simulink model "AirDirecCtrlMgr"  (R2022b) (continued)
== system AirDirecCtrlMgr == (continued)
  ClimaCfgForVgaMotorPrsntAtRowFirstSideLe_ClimaCfgForVgaMotorPrsntAtRowFirstSideLe/1 -> AirDirecCtrlMgr_Runnable_sys/4(ClimaCfgForVgaMotorPrsntAtRowFirstSideLe_ClimaCfgForVgaMotorPrsntAtRowFirstSideLe_read)
  ClimaCfgForVgaMotorPrsntAtRowFirstSideRi_ClimaCfgForVgaMotorPrsntAtRowFirstSideRi/1 -> AirDirecCtrlMgr_Runnable_sys/5(ClimaCfgForVgaMotorPrsntAtRowFirstSideRi_ClimaCfgForVgaMotorPrsntAtRowFirstSideRi_read)
  ClimaCfgForVgaMotorPrsntAtRowSecSideLe_ClimaCfgForVgaMotorPrsntAtRowSecSideLe/1 -> AirDirecCtrlMgr_Runnable_sys/6(ClimaCfgForVgaMotorPrsntAtRowSecSideLe_ClimaCfgForVgaMotorPrsntAtRowSecSideLe_read)
  ClimaCfgForVgaMotorPrsntAtRowSecSideRi_ClimaCfgForVgaMotorPrsntAtRowSecSideRi/1 -> AirDirecCtrlMgr_Runnable_sys/7(ClimaCfgForVgaMotorPrsntAtRowSecSideRi_ClimaCfgForVgaMotorPrsntAtRowSecSideRi_read)
  ClimaCtrlActvNgcc_ClimaCtrlActv/1 -> AirDirecCtrlMgr_Runnable_sys/8(ClimaCtrlActvNgcc_ClimaCtrlActv_read)
  DrvrHdPosnDetn_DrvrHdPosnDetn/1 -> AirDirecCtrlMgr_Runnable_sys/9(DrvrHdPosnDetn_DrvrHdPosnDetn_read)
  FrntHvacBlowerSts_FrntHvacBlowerSts/1 -> AirDirecCtrlMgr_Runnable_sys/10(FrntHvacBlowerSts_FrntHvacBlowerSts_read)
  FrntVGAerror_FrntVGAerror/1 -> AirDirecCtrlMgr_Runnable_sys/11(FrntVGAerror_FrntVGAerror_read)
  HmiElecAirAdpvReq_HmiElecAirAdpvReq/1 -> AirDirecCtrlMgr_Runnable_sys/12(HmiElecAirAdpvReq_HmiElecAirAdpvReq_read)
  HmiElecAirDirCrtlReq_HmiElecAirDirCrtlReq/1 -> AirDirecCtrlMgr_Runnable_sys/13(HmiElecAirDirCrtlReq_HmiElecAirDirCrtlReq_read)
  HmiElecAirDirModReq_HmiElecAirDirModReq/1 -> AirDirecCtrlMgr_Runnable_sys/14(HmiElecAirDirModReq_HmiElecAirDirModReq_read)
  HmiElecAirDrvrSwtReq_HmiElecAirDrvrSwtReq/1 -> AirDirecCtrlMgr_Runnable_sys/15(HmiElecAirDrvrSwtReq_HmiElecAirDrvrSwtReq_read)
  HmiElecAirFrntSwtReq_HmiElecAirFrntSwtReq/1 -> AirDirecCtrlMgr_Runnable_sys/16(HmiElecAirFrntSwtReq_HmiElecAirFrntSwtReq_read)
  HmiElecAirPassSwtReq_HmiElecAirPassSwtReq/1 -> AirDirecCtrlMgr_Runnable_sys/17(HmiElecAirPassSwtReq_HmiElecAirPassSwtReq_read)
  HmiElecAirReSwtReq_HmiElecAirReSwtReq/1 -> AirDirecCtrlMgr_Runnable_sys/18(HmiElecAirReSwtReq_HmiElecAirReSwtReq_read)
  HmiElecAirSwngReq_HmiElecAirSwngReq/1 -> AirDirecCtrlMgr_Runnable_sys/19(HmiElecAirSwngReq_HmiElecAirSwngReq_read)
  HmiReElecAirDirCrtlReq_HmiReElecAirDirCrtlReq/1 -> AirDirecCtrlMgr_Runnable_sys/20(HmiReElecAirDirCrtlReq_HmiReElecAirDirCrtlReq_read)
  RearVGAerror_RearVGAerror/1 -> AirDirecCtrlMgr_Runnable_sys/21(RearVGAerror_RearVGAerror_read)
  ResrvdSigForECM4_ResrvdSigForECM4/1 -> AirDirecCtrlMgr_Runnable_sys/22(ResrvdSigForECM4_ResrvdSigForECM4_read)
  StrtRemSts1WdStrtRemSts_Ngcc_StrtRemSts1WdStrtRemSts/1 -> AirDirecCtrlMgr_Runnable_sys/23(StrtRemSts1WdStrtRemSts_Ngcc_StrtRemSts1WdStrtRemSts_read)
  VehModMngtGlbSafe1_Ngcc_VehModMngtGlbSafe1/1 -> AirDirecCtrlMgr_Runnable_sys/24(VehModMngtGlbSafe1_Ngcc_VehModMngtGlbSafe1_read)
  VentnDrvrLeXStsRsp_VentnDrvrLeXStsRsp/1 -> AirDirecCtrlMgr_Runnable_sys/25(VentnDrvrLeXStsRsp_VentnDrvrLeXStsRsp_read)
  VentnDrvrLeYStsRsp_VentnDrvrLeYStsRsp/1 -> AirDirecCtrlMgr_Runnable_sys/26(VentnDrvrLeYStsRsp_VentnDrvrLeYStsRsp_read)
  VentnDrvrRiXStsRsp_VentnDrvrRiXStsRsp/1 -> AirDirecCtrlMgr_Runnable_sys/27(VentnDrvrRiXStsRsp_VentnDrvrRiXStsRsp_read)
  VentnDrvrRiYStsRsp_VentnDrvrRiYStsRsp/1 -> AirDirecCtrlMgr_Runnable_sys/28(VentnDrvrRiYStsRsp_VentnDrvrRiYStsRsp_read)
  VentnPassLeXStsRsp_VentnPassLeXStsRsp/1 -> AirDirecCtrlMgr_Runnable_sys/29(VentnPassLeXStsRsp_VentnPassLeXStsRsp_read)
  VentnPassLeYStsRsp_VentnPassLeYStsRsp/1 -> AirDirecCtrlMgr_Runnable_sys/30(VentnPassLeYStsRsp_VentnPassLeYStsRsp_read)
  VentnPassRiXStsRsp_VentnPassRiXStsRsp/1 -> AirDirecCtrlMgr_Runnable_sys/31(VentnPassRiXStsRsp_VentnPassRiXStsRsp_read)
  VentnPassRiYStsRsp_VentnPassRiYStsRsp/1 -> AirDirecCtrlMgr_Runnable_sys/32(VentnPassRiYStsRsp_VentnPassRiYStsRsp_read)
  VentnSecLeXStsRsp_VentnSecLeXStsRsp/1 -> AirDirecCtrlMgr_Runnable_sys/33(VentnSecLeXStsRsp_VentnSecLeXStsRsp_read)
  VentnSecLeYStsRsp_VentnSecLeYStsRsp/1 -> AirDirecCtrlMgr_Runnable_sys/34(VentnSecLeYStsRsp_VentnSecLeYStsRsp_read)
  VentnSecRiXStsRsp_VentnSecRiXStsRsp/1 -> AirDirecCtrlMgr_Runnable_sys/35(VentnSecRiXStsRsp_VentnSecRiXStsRsp_read)
  VentnSecRiYStsRsp_VentnSecRiYStsRsp/1 -> AirDirecCtrlMgr_Runnable_sys/36(VentnSecRiYStsRsp_VentnSecRiYStsRsp_read)
  AmbTFildForClimaNgcc_AmbTFildForClima/1 -> AirDirecCtrlMgr_Runnable_sys/37(AmbTFildForClimaNgcc_AmbTFildForClima_read)
  BlwAftRunSts_BlwAftRunSts/1 -> AirDirecCtrlMgr_Runnable_sys/38(BlwAftRunSts_BlwAftRunSts_read)
  CcmElecAirDirCrtlReqDrvrLePosX_CcmElecAirDirCrtlReqDrvrLePosX/1 -> AirDirecCtrlMgr_Runnable_sys/39(CcmElecAirDirCrtlReqDrvrLePosX_CcmElecAirDirCrtlReqDrvrLePosX_read)
  CcmElecAirDirCrtlReqDrvrLePosY_CcmElecAirDirCrtlReqDrvrLePosY/1 -> AirDirecCtrlMgr_Runnable_sys/40(CcmElecAirDirCrtlReqDrvrLePosY_CcmElecAirDirCrtlReqDrvrLePosY_read)
  CcmElecAirDirCrtlReqDrvrRiPosX_CcmElecAirDirCrtlReqDrvrRiPosX/1 -> AirDirecCtrlMgr_Runnable_sys/41(CcmElecAirDirCrtlReqDrvrRiPosX_CcmElecAirDirCrtlReqDrvrRiPosX_read)
  CcmElecAirDirCrtlReqDrvrRiPosY_CcmElecAirDirCrtlReqDrvrRiPosY/1 -> AirDirecCtrlMgr_Runnable_sys/42(CcmElecAirDirCrtlReqDrvrRiPosY_CcmElecAirDirCrtlReqDrvrRiPosY_read)
  CcmElecAirDirCrtlReqPassLePosX_CcmElecAirDirCrtlReqPassLePosX/1 -> AirDirecCtrlMgr_Runnable_sys/43(CcmElecAirDirCrtlReqPassLePosX_CcmElecAirDirCrtlReqPassLePosX_read)
  CcmElecAirDirCrtlReqPassLePosY_CcmElecAirDirCrtlReqPassLePosY/1 -> AirDirecCtrlMgr_Runnable_sys/44(CcmElecAirDirCrtlReqPassLePosY_CcmElecAirDirCrtlReqPassLePosY_read)
  CcmElecAirDirCrtlReqPassRiPosX_CcmElecAirDirCrtlReqPassRiPosX/1 -> AirDirecCtrlMgr_Runnable_sys/45(CcmElecAirDirCrtlReqPassRiPosX_CcmElecAirDirCrtlReqPassRiPosX_read)
  CcmElecAirDirCrtlReqPassRiPosY_CcmElecAirDirCrtlReqPassRiPosY/1 -> AirDirecCtrlMgr_Runnable_sys/46(CcmElecAirDirCrtlReqPassRiPosY_CcmElecAirDirCrtlReqPassRiPosY_read)
  ClimaCfgForHeadRadar_ClimaCfgForHeadRadar/1 -> AirDirecCtrlMgr_Runnable_sys/47(ClimaCfgForHeadRadar_ClimaCfgForHeadRadar_read)
  ClimaTAtCmftForRowFirstLe_ClimaTAtCmftForRowFirstLe/1 -> AirDirecCtrlMgr_Runnable_sys/48(ClimaTAtCmftForRowFirstLe_ClimaTAtCmftForRowFirstLe_read)
  ClimaTAtCmftForRowFirstRi_ClimaTAtCmftForRowFirstRi/1 -> AirDirecCtrlMgr_Runnable_sys/49(ClimaTAtCmftForRowFirstRi_ClimaTAtCmftForRowFirstRi_read)
  ClimaTAtCmftForRowSecLe_ClimaTAtCmftForRowSecLe/1 -> AirDirecCtrlMgr_Runnable_sys/50(ClimaTAtCmftForRowSecLe_ClimaTAtCmftForRowSecLe_read)
  ClimaTAtCmftForRowSecRi_ClimaTAtCmftForRowSecRi/1 -> AirDirecCtrlMgr_Runnable_sys/51(ClimaTAtCmftForRowSecRi_ClimaTAtCmftForRowSecRi_read)
  CmptmtAirTCtrlErrAtRowFirstAvg_CmptmtAirTCtrlErrAtRowFirstAvg/1 -> AirDirecCtrlMgr_Runnable_sys/52(CmptmtAirTCtrlErrAtRowFirstAvg_CmptmtAirTCtrlErrAtRowFirstAvg_read)
  CmptmtAirTCtrlErrAtRowFirstLe_CmptmtAirTCtrlErrAtRowFirstLe/1 -> AirDirecCtrlMgr_Runnable_sys/53(CmptmtAirTCtrlErrAtRowFirstLe_CmptmtAirTCtrlErrAtRowFirstLe_read)
  CmptmtAirTCtrlErrAtRowFirstRi_CmptmtAirTCtrlErrAtRowFirstRi/1 -> AirDirecCtrlMgr_Runnable_sys/54(CmptmtAirTCtrlErrAtRowFirstRi_CmptmtAirTCtrlErrAtRowFirstRi_read)
  CmptmtAirTEstimdAtRowFirst_CmptmtAirTEstimdAtRowFirst/1 -> AirDirecCtrlMgr_Runnable_sys/55(CmptmtAirTEstimdAtRowFirst_CmptmtAirTEstimdAtRowFirst_read)
  CmptmtClngActv_CmptmtClngActv/1 -> AirDirecCtrlMgr_Runnable_sys/56(CmptmtClngActv_CmptmtClngActv_read)
  CmptmtIntrMtrlTErr_CmptmtIntrMtrlTErr/1 -> AirDirecCtrlMgr_Runnable_sys/57(CmptmtIntrMtrlTErr_CmptmtIntrMtrlTErr_read)
  CmptmtIntrMtrlTEstimd_CmptmtIntrMtrlTEstimd/1 -> AirDirecCtrlMgr_Runnable_sys/58(CmptmtIntrMtrlTEstimd_CmptmtIntrMtrlTEstimd_read)
  CmptmtSunMtrlTErr_CmptmtSunMtrlTErr/1 -> AirDirecCtrlMgr_Runnable_sys/59(CmptmtSunMtrlTErr_CmptmtSunMtrlTErr_read)
  CmptmtSunMtrlTEstimd_CmptmtSunMtrlTEstimd/1 -> AirDirecCtrlMgr_Runnable_sys/60(CmptmtSunMtrlTEstimd_CmptmtSunMtrlTEstimd_read)
  CmptmtTFrntRaw_CmptmtTFrntRaw/1 -> AirDirecCtrlMgr_Runnable_sys/61(CmptmtTFrntRaw_CmptmtTFrntRaw_read)
  DrvrPfmncAlrmReq_DrvrPfmncAlrmReq/1 -> AirDirecCtrlMgr_Runnable_sys/62(DrvrPfmncAlrmReq_DrvrPfmncAlrmReq_read)
  DrvrPfmncAlrmReqFromDHU_DrvrPfmncAlrmReqFromDHU/1 -> AirDirecCtrlMgr_Runnable_sys/63(DrvrPfmncAlrmReqFromDHU_DrvrPfmncAlrmReqFromDHU_read)
  FbForCmptmtT_FbForCmptmtT/1 -> AirDirecCtrlMgr_Runnable_sys/64(FbForCmptmtT_FbForCmptmtT_read)
  FragStsFrmClima_FragStsFrmClima/1 -> AirDirecCtrlMgr_Runnable_sys/65(FragStsFrmClima_FragStsFrmClima_read)
  HmiCmptmtAirDistbnFrntForClima_HmiCmptmtAirDistbnFrntForClima/1 -> AirDirecCtrlMgr_Runnable_sys/66(HmiCmptmtAirDistbnFrntForClima_HmiCmptmtAirDistbnFrntForClima_read)
  HmiCmptmtAirDistbnFrntForClima_HmiCmptmtAirDistbnFrntRiForClima/1 -> AirDirecCtrlMgr_Runnable_sys/67(HmiCmptmtAirDistbnFrntForClima_HmiCmptmtAirDistbnFrntRiForClima_read)
  HmiCmptmtAirDistbnFrntForClima_HmiCmptmtAirDistbnReForClima/1 -> AirDirecCtrlMgr_Runnable_sys/68(HmiCmptmtAirDistbnFrntForClima_HmiCmptmtAirDistbnReForClima_read)
  HmiCmptmtAirDistbnFrntForClima_HmiCmptmtAirDistbnReRiForClima/1 -> AirDirecCtrlMgr_Runnable_sys/69(HmiCmptmtAirDistbnFrntForClima_HmiCmptmtAirDistbnReRiForClima_read)
  HmiCmptmtTSpForClima_HmiCmptmtTSpForClima/1 -> AirDirecCtrlMgr_Runnable_sys/70(HmiCmptmtTSpForClima_HmiCmptmtTSpForClima_read)
  HvacFlowFrntActForRowFirst_HvacFlowFrntActForRowFirst/1 -> AirDirecCtrlMgr_Runnable_sys/71(HvacFlowFrntActForRowFirst_HvacFlowFrntActForRowFirst_read)
  HvacFlowFrntActForRowFirstAtVent_HvacFlowFrntActForRowFirstAtVent/1 -> AirDirecCtrlMgr_Runnable_sys/72(HvacFlowFrntActForRowFirstAtVent_HvacFlowFrntActForRowFirstAtVent_read)
  HvacFlowFrntActForRowFirstAtVent_HvacFlowFrntActForRowFirstAtVentLe/1 -> AirDirecCtrlMgr_Runnable_sys/73(HvacFlowFrntActForRowFirstAtVent_HvacFlowFrntActForRowFirstAtVentLe_read)
  HvacFlowFrntActForRowFirstAtVent_HvacFlowFrntActForRowFirstAtVentRi/1 -> AirDirecCtrlMgr_Runnable_sys/74(HvacFlowFrntActForRowFirstAtVent_HvacFlowFrntActForRowFirstAtVentRi_read)
  HvacFlowFrntActForRowFirstAtVent_HvacFlowFrntActForRowFirstAtVentSideLe/1 -> AirDirecCtrlMgr_Runnable_sys/75(HvacFlowFrntActForRowFirstAtVent_HvacFlowFrntActForRowFirstAtVentSideLe_read)
  HvacFlowFrntActForRowFirstAtVent_HvacFlowFrntActForRowFirstAtVentSideRi/1 -> AirDirecCtrlMgr_Runnable_sys/76(HvacFlowFrntActForRowFirstAtVent_HvacFlowFrntActForRowFirstAtVentSideRi_read)
  HvacFlowFrntActForRowSec_HvacFlowFrntActForRowSec/1 -> AirDirecCtrlMgr_Runnable_sys/77(HvacFlowFrntActForRowSec_HvacFlowFrntActForRowSec_read)
  HvacFlowFrntActForRowSecAtVent_HvacFlowFrntActForRowSecAtVent/1 -> AirDirecCtrlMgr_Runnable_sys/78(HvacFlowFrntActForRowSecAtVent_HvacFlowFrntActForRowSecAtVent_read)
  HvacFlowFrntActForRowSecAtVent_HvacFlowFrntActForRowSecAtVentLe/1 -> AirDirecCtrlMgr_Runnable_sys/79(HvacFlowFrntActForRowSecAtVent_HvacFlowFrntActForRowSecAtVentLe_read)
  HvacFlowFrntActForRowSecAtVent_HvacFlowFrntActForRowSecAtVentRi/1 -> AirDirecCtrlMgr_Runnable_sys/80(HvacFlowFrntActForRowSecAtVent_HvacFlowFrntActForRowSecAtVentRi_read)
  HvacFlowFrntForRowFirstAtVentLe_HvacFlowFrntForRowFirstAtVentLe/1 -> AirDirecCtrlMgr_Runnable_sys/81(HvacFlowFrntForRowFirstAtVentLe_HvacFlowFrntForRowFirstAtVentLe_read)
  HvacFlowFrntForRowFirstAtVentRi_HvacFlowFrntForRowFirstAtVentRi/1 -> AirDirecCtrlMgr_Runnable_sys/82(HvacFlowFrntForRowFirstAtVentRi_HvacFlowFrntForRowFirstAtVentRi_read)
  HvacFlowFrntForRowFirstAtVentSideLe_HvacFlowFrntForRowFirstAtVentSideLe/1 -> AirDirecCtrlMgr_Runnable_sys/83(HvacFlowFrntForRowFirstAtVentSideLe_HvacFlowFrntForRowFirstAtVentSideLe_read)
  HvacFlowFrntForRowFirstAtVentSideRi_HvacFlowFrntForRowFirstAtVentSideRi/1 -> AirDirecCtrlMgr_Runnable_sys/84(HvacFlowFrntForRowFirstAtVentSideRi_HvacFlowFrntForRowFirstAtVentSideRi_read)
  HvacTFrntForRowFirstAtVentLe_HvacTFrntForRowFirstAtVentLe/1 -> AirDirecCtrlMgr_Runnable_sys/85(HvacTFrntForRowFirstAtVentLe_HvacTFrntForRowFirstAtVentLe_read)
  HvacTFrntForRowFirstAtVentRi_HvacTFrntForRowFirstAtVentRi/1 -> AirDirecCtrlMgr_Runnable_sys/86(HvacTFrntForRowFirstAtVentRi_HvacTFrntForRowFirstAtVentRi_read)
  HvacTFrntForRowFirstAtVentSideLe_HvacTFrntForRowFirstAtVentSideLe/1 -> AirDirecCtrlMgr_Runnable_sys/87(HvacTFrntForRowFirstAtVentSideLe_HvacTFrntForRowFirstAtVentSideLe_read)
  HvacTFrntForRowFirstAtVentSideRi_HvacTFrntForRowFirstAtVentSideRi/1 -> AirDirecCtrlMgr_Runnable_sys/88(HvacTFrntForRowFirstAtVentSideRi_HvacTFrntForRowFirstAtVentSideRi_read)
  HvacTFrntForRowSecAtVentLe_HvacTFrntForRowSecAtVentLe/1 -> AirDirecCtrlMgr_Runnable_sys/89(HvacTFrntForRowSecAtVentLe_HvacTFrntForRowSecAtVentLe_read)
  HvacTFrntForRowSecAtVentRi_HvacTFrntForRowSecAtVentRi/1 -> AirDirecCtrlMgr_Runnable_sys/90(HvacTFrntForRowSecAtVentRi_HvacTFrntForRowSecAtVentRi_read)
  PassSideHdPosnDetn_PassSideHdPosnDetn/1 -> AirDirecCtrlMgr_Runnable_sys/91(PassSideHdPosnDetn_PassSideHdPosnDetn_read)
  PrkgClimaOnReq_PrkgClimaOnReq/1 -> AirDirecCtrlMgr_Runnable_sys/92(PrkgClimaOnReq_PrkgClimaOnReq_read)
  PwrAllwdForElecAirVentn_Ngcc_PwrAllwdForElecAirVentn/1 -> AirDirecCtrlMgr_Runnable_sys/93(PwrAllwdForElecAirVentn_Ngcc_PwrAllwdForElecAirVentn_read)
  RemVentActvSts_RemVentActvSts/1 -> AirDirecCtrlMgr_Runnable_sys/94(RemVentActvSts_RemVentActvSts_read)
  SunData_SunData/1 -> AirDirecCtrlMgr_Runnable_sys/95(SunData_SunData_read)
  VehSpdLgtForClima_VehSpdLgtForClima/1 -> AirDirecCtrlMgr_Runnable_sys/96(VehSpdLgtForClima_VehSpdLgtForClima_read)
  VehSunLoadAtRowFirstLeFild_VehSunLoadAtRowFirstLeFild/1 -> AirDirecCtrlMgr_Runnable_sys/97(VehSunLoadAtRowFirstLeFild_VehSunLoadAtRowFirstLeFild_read)
  VehSunLoadAtRowFirstRiFild_VehSunLoadAtRowFirstRiFild/1 -> AirDirecCtrlMgr_Runnable_sys/98(VehSunLoadAtRowFirstRiFild_VehSunLoadAtRowFirstRiFild_read)
  VehSunLoadTot_VehSunLoadTot/1 -> AirDirecCtrlMgr_Runnable_sys/99(VehSunLoadTot_VehSunLoadTot_read)
  ClimaCfgForElectricLiftingModule_ClimaCfgForElectricLiftingModule/1 -> AirDirecCtrlMgr_Runnable_sys/100(ClimaCfgForElectricLiftingModule_ClimaCfgForElectricLiftingModule_read)
  ClimaCfgForInteriorViewCamera_ClimaCfgForInteriorViewCamera/1 -> AirDirecCtrlMgr_Runnable_sys/101(ClimaCfgForInteriorViewCamera_ClimaCfgForInteriorViewCamera_read)
  ClimaCfgForMARKET_ClimaCfgForMARKET/1 -> AirDirecCtrlMgr_Runnable_sys/102(ClimaCfgForMARKET_ClimaCfgForMARKET_read)
  ClimaCfgForPowerSeats_ClimaCfgForPowerSeats/1 -> AirDirecCtrlMgr_Runnable_sys/103(ClimaCfgForPowerSeats_ClimaCfgForPowerSeats_read)
  ClimaCfgForVgaMotor1_ClimaCfgForVgaMotor1/1 -> AirDirecCtrlMgr_Runnable_sys/104(ClimaCfgForVgaMotor1_ClimaCfgForVgaMotor1_read)
  ClimaCfgForVgaMotor10_ClimaCfgForVgaMotor10/1 -> AirDirecCtrlMgr_Runnable_sys/105(ClimaCfgForVgaMotor10_ClimaCfgForVgaMotor10_read)
  ClimaCfgForVgaMotor11_ClimaCfgForVgaMotor11/1 -> AirDirecCtrlMgr_Runnable_sys/106(ClimaCfgForVgaMotor11_ClimaCfgForVgaMotor11_read)
  ClimaCfgForVgaMotor12_ClimaCfgForVgaMotor12/1 -> AirDirecCtrlMgr_Runnable_sys/107(ClimaCfgForVgaMotor12_ClimaCfgForVgaMotor12_read)
  ClimaCfgForVgaMotor13_ClimaCfgForVgaMotor13/1 -> AirDirecCtrlMgr_Runnable_sys/108(ClimaCfgForVgaMotor13_ClimaCfgForVgaMotor13_read)
  ClimaCfgForVgaMotor14_ClimaCfgForVgaMotor14/1 -> AirDirecCtrlMgr_Runnable_sys/109(ClimaCfgForVgaMotor14_ClimaCfgForVgaMotor14_read)
  ClimaCfgForVgaMotor15_ClimaCfgForVgaMotor15/1 -> AirDirecCtrlMgr_Runnable_sys/110(ClimaCfgForVgaMotor15_ClimaCfgForVgaMotor15_read)
  ClimaCfgForVgaMotor16_ClimaCfgForVgaMotor16/1 -> AirDirecCtrlMgr_Runnable_sys/111(ClimaCfgForVgaMotor16_ClimaCfgForVgaMotor16_read)
  ClimaCfgForVgaMotor17_ClimaCfgForVgaMotor17/1 -> AirDirecCtrlMgr_Runnable_sys/112(ClimaCfgForVgaMotor17_ClimaCfgForVgaMotor17_read)
  ClimaCfgForVgaMotor18_ClimaCfgForVgaMotor18/1 -> AirDirecCtrlMgr_Runnable_sys/113(ClimaCfgForVgaMotor18_ClimaCfgForVgaMotor18_read)
  ClimaCfgForVgaMotor19_ClimaCfgForVgaMotor19/1 -> AirDirecCtrlMgr_Runnable_sys/114(ClimaCfgForVgaMotor19_ClimaCfgForVgaMotor19_read)
  ClimaCfgForVgaMotor2_ClimaCfgForVgaMotor2/1 -> AirDirecCtrlMgr_Runnable_sys/115(ClimaCfgForVgaMotor2_ClimaCfgForVgaMotor2_read)
  ClimaCfgForVgaMotor20_ClimaCfgForVgaMotor20/1 -> AirDirecCtrlMgr_Runnable_sys/116(ClimaCfgForVgaMotor20_ClimaCfgForVgaMotor20_read)
  ClimaCfgForVgaMotor3_ClimaCfgForVgaMotor3/1 -> AirDirecCtrlMgr_Runnable_sys/117(ClimaCfgForVgaMotor3_ClimaCfgForVgaMotor3_read)
  ClimaCfgForVgaMotor4_ClimaCfgForVgaMotor4/1 -> AirDirecCtrlMgr_Runnable_sys/118(ClimaCfgForVgaMotor4_ClimaCfgForVgaMotor4_read)
  ClimaCfgForVgaMotor5_ClimaCfgForVgaMotor5/1 -> AirDirecCtrlMgr_Runnable_sys/119(ClimaCfgForVgaMotor5_ClimaCfgForVgaMotor5_read)
  ClimaCfgForVgaMotor6_ClimaCfgForVgaMotor6/1 -> AirDirecCtrlMgr_Runnable_sys/120(ClimaCfgForVgaMotor6_ClimaCfgForVgaMotor6_read)
  ClimaCfgForVgaMotor7_ClimaCfgForVgaMotor7/1 -> AirDirecCtrlMgr_Runnable_sys/121(ClimaCfgForVgaMotor7_ClimaCfgForVgaMotor7_read)
  ClimaCfgForVgaMotor8_ClimaCfgForVgaMotor8/1 -> AirDirecCtrlMgr_Runnable_sys/122(ClimaCfgForVgaMotor8_ClimaCfgForVgaMotor8_read)
  ClimaCfgForVgaMotor9_ClimaCfgForVgaMotor9/1 -> AirDirecCtrlMgr_Runnable_sys/123(ClimaCfgForVgaMotor9_ClimaCfgForVgaMotor9_read)
  ClimaCfgWindFollowHumanMov_ClimaCfgWindFollowHumanMov/1 -> AirDirecCtrlMgr_Runnable_sys/124(ClimaCfgWindFollowHumanMov_ClimaCfgWindFollowHumanMov_read)
  CmptmtAirTCtrlErrQf_CmptmtAirTCtrlErrQf/1 -> AirDirecCtrlMgr_Runnable_sys/125(CmptmtAirTCtrlErrQf_CmptmtAirTCtrlErrQf_read)
  CopilotSeatOccupySts_CopilotSeatOccupySts/1 -> AirDirecCtrlMgr_Runnable_sys/126(CopilotSeatOccupySts_CopilotSeatOccupySts_read)
  CopilotSeatOccupySts_CopilotSeatOccupySts_IsUpdated/1 -> AirDirecCtrlMgr_Runnable_sys/127(CopilotSeatOccupySts_CopilotSeatOccupySts_IsUpdated_read)
  DrvrSeatPosPercNgcc_DrvrSeatPosPercNgcc/1 -> AirDirecCtrlMgr_Runnable_sys/128(DrvrSeatPosPercNgcc_DrvrSeatPosPercNgcc_read)
  DrvrSeatPosPercNgcc_DrvrSeatPosPercNgcc_IsUpdated/1 -> AirDirecCtrlMgr_Runnable_sys/129(DrvrSeatPosPercNgcc_DrvrSeatPosPercNgcc_IsUpdated_read)
  DrvSeatOccupySts_DrvSeatOccupySts/1 -> AirDirecCtrlMgr_Runnable_sys/130(DrvSeatOccupySts_DrvSeatOccupySts_read)
  DrvSeatOccupySts_DrvSeatOccupySts_IsUpdated/1 -> AirDirecCtrlMgr_Runnable_sys/131(DrvSeatOccupySts_DrvSeatOccupySts_IsUpdated_read)
  HmiElecAirBodyVentLebtnNgcc_HmiElecAirBodyVentLebtnNgcc/1 -> AirDirecCtrlMgr_Runnable_sys/132(HmiElecAirBodyVentLebtnNgcc_HmiElecAirBodyVentLebtnNgcc_read)
  HmiElecAirBodyVentRibtnNgcc_HmiElecAirBodyVentRibtnNgcc/1 -> AirDirecCtrlMgr_Runnable_sys/133(HmiElecAirBodyVentRibtnNgcc_HmiElecAirBodyVentRibtnNgcc_read)
  HmiMaxACReq_HmiMaxACReq/1 -> AirDirecCtrlMgr_Runnable_sys/134(HmiMaxACReq_HmiMaxACReq_read)
  HmiSmrtWindFolwSetNgcc_HmiSmrtWindFolwSetNgcc/1 -> AirDirecCtrlMgr_Runnable_sys/135(HmiSmrtWindFolwSetNgcc_HmiSmrtWindFolwSetNgcc_read)
  PassSeatPosPercNgcc_PassSeatPosPercNgcc/1 -> AirDirecCtrlMgr_Runnable_sys/136(PassSeatPosPercNgcc_PassSeatPosPercNgcc_read)
  PassSeatPosPercNgcc_PassSeatPosPercNgcc_IsUpdated/1 -> AirDirecCtrlMgr_Runnable_sys/137(PassSeatPosPercNgcc_PassSeatPosPercNgcc_IsUpdated_read)
  SeatBackAngleRowFirstDrvrNgcc_SeatBackAngleRowFirstDrvrNgcc/1 -> AirDirecCtrlMgr_Runnable_sys/138(SeatBackAngleRowFirstDrvrNgcc_SeatBackAngleRowFirstDrvrNgcc_read)
  SeatBackAngleRowFirstDrvrNgcc_SeatBackAngleRowFirstDrvrNgcc_IsUpdated/1 -> AirDirecCtrlMgr_Runnable_sys/139(SeatBackAngleRowFirstDrvrNgcc_SeatBackAngleRowFirstDrvrNgcc_IsUpdated_read)
  SeatBackAngleRowFirstPassNgcc_SeatBackAngleRowFirstPassNgcc/1 -> AirDirecCtrlMgr_Runnable_sys/140(SeatBackAngleRowFirstPassNgcc_SeatBackAngleRowFirstPassNgcc_read)
  SeatBackAngleRowFirstPassNgcc_SeatBackAngleRowFirstPassNgcc_IsUpdated/1 -> AirDirecCtrlMgr_Runnable_sys/141(SeatBackAngleRowFirstPassNgcc_SeatBackAngleRowFirstPassNgcc_IsUpdated_read)
  SeatOccptAtRowSecLe_SeatOccptAtRowSecLe/1 -> AirDirecCtrlMgr_Runnable_sys/142(SeatOccptAtRowSecLe_SeatOccptAtRowSecLe_read)
  SeatOccptAtRowSecLe_SeatOccptAtRowSecLe_IsUpdated/1 -> AirDirecCtrlMgr_Runnable_sys/143(SeatOccptAtRowSecLe_SeatOccptAtRowSecLe_IsUpdated_read)
  SeatOccptAtRowSecMid_SeatOccptAtRowSecMid/1 -> AirDirecCtrlMgr_Runnable_sys/144(SeatOccptAtRowSecMid_SeatOccptAtRowSecMid_read)
  SeatOccptAtRowSecMid_SeatOccptAtRowSecMid_IsUpdated/1 -> AirDirecCtrlMgr_Runnable_sys/145(SeatOccptAtRowSecMid_SeatOccptAtRowSecMid_IsUpdated_read)
  SeatOccptAtRowSecRi_SeatOccptAtRowSecRi/1 -> AirDirecCtrlMgr_Runnable_sys/146(SeatOccptAtRowSecRi_SeatOccptAtRowSecRi_read)
  SeatOccptAtRowSecRi_SeatOccptAtRowSecRi_IsUpdated/1 -> AirDirecCtrlMgr_Runnable_sys/147(SeatOccptAtRowSecRi_SeatOccptAtRowSecRi_IsUpdated_read)
  TiAndDateIndcn_TiAndDateIndcn/1 -> AirDirecCtrlMgr_Runnable_sys/148(TiAndDateIndcn_TiAndDateIndcn_read)
  VentnLiftModPosnRsp_VentnLiftModPosnRsp/1 -> AirDirecCtrlMgr_Runnable_sys/149(VentnLiftModPosnRsp_VentnLiftModPosnRsp_read)
  VentnLiftModStsRsp_VentnLiftModStsRsp/1 -> AirDirecCtrlMgr_Runnable_sys/150(VentnLiftModStsRsp_VentnLiftModStsRsp_read)
  AirDirecCtrlMgr_Runnable_sys/1(DrvrVentLeClsdFlag_DrvrVentLeClsdFlag_write) -> DrvrVentLeClsdFlag_DrvrVentLeClsdFlag/1
  AirDirecCtrlMgr_Runnable_sys/2(DrvrVentRiClsdFlag_DrvrVentRiClsdFlag_write) -> DrvrVentRiClsdFlag_DrvrVentRiClsdFlag/1
  AirDirecCtrlMgr_Runnable_sys/3(MemSaveFlgForCenVentLiftModErr_MemSaveFlgForCenVentLiftModErr_write) -> MemSaveFlgForCenVentLiftModErr_MemSaveFlgForCenVentLiftModErr/1
  AirDirecCtrlMgr_Runnable_sys/4(MemSaveForCenVentLiftModSts_MemSaveForCenVentLiftModSts_write) -> MemSaveForCenVentLiftModSts_MemSaveForCenVentLiftModSts/1
  AirDirecCtrlMgr_Runnable_sys/5(PassVentLeClsdFlag_PassVentLeClsdFlag_write) -> PassVentLeClsdFlag_PassVentLeClsdFlag/1
  AirDirecCtrlMgr_Runnable_sys/6(PassVentRiClsdFlag_PassVentRiClsdFlag_write) -> PassVentRiClsdFlag_PassVentRiClsdFlag/1
  AirDirecCtrlMgr_Runnable_sys/7(VentLiftModCaliReq_VentLiftModCaliReq_write) -> VentLiftModCaliReq_VentLiftModCaliReq/1
  AirDirecCtrlMgr_Runnable_sys/8(VentnDrvrLeXPsonReq_VentnDrvrLeXPsonReq_write) -> VentnDrvrLeXPsonReq_VentnDrvrLeXPsonReq/1
  AirDirecCtrlMgr_Runnable_sys/9(VentnDrvrLeYPsonReq_VentnDrvrLeYPsonReq_write) -> VentnDrvrLeYPsonReq_VentnDrvrLeYPsonReq/1
  AirDirecCtrlMgr_Runnable_sys/10(VentnDrvrRiXPsonReq_VentnDrvrRiXPsonReq_write) -> VentnDrvrRiXPsonReq_VentnDrvrRiXPsonReq/1
  AirDirecCtrlMgr_Runnable_sys/11(VentnDrvrRiYPsonReq_VentnDrvrRiYPsonReq_write) -> VentnDrvrRiYPsonReq_VentnDrvrRiYPsonReq/1
  AirDirecCtrlMgr_Runnable_sys/12(VentnLiftModPosnReq_VentnLiftModPosnReq_write) -> VentnLiftModPosnReq_VentnLiftModPosnReq/1
  AirDirecCtrlMgr_Runnable_sys/13(VentnPassLeXPsonReq_VentnPassLeXPsonReq_write) -> VentnPassLeXPsonReq_VentnPassLeXPsonReq/1
  AirDirecCtrlMgr_Runnable_sys/14(VentnPassLeYPsonReq_VentnPassLeYPsonReq_write) -> VentnPassLeYPsonReq_VentnPassLeYPsonReq/1
  AirDirecCtrlMgr_Runnable_sys/15(VentnPassRiXPsonReq_VentnPassRiXPsonReq_write) -> VentnPassRiXPsonReq_VentnPassRiXPsonReq/1
  AirDirecCtrlMgr_Runnable_sys/16(VentnPassRiYPsonReq_VentnPassRiYPsonReq_write) -> VentnPassRiYPsonReq_VentnPassRiYPsonReq/1
  AirDirecCtrlMgr_Runnable_sys/17(VentnSecLeXPosnReq_VentnSecLeXPosnReq_write) -> VentnSecLeXPosnReq_VentnSecLeXPosnReq/1
  AirDirecCtrlMgr_Runnable_sys/18(VentnSecLeYPosnReq_VentnSecLeYPosnReq_write) -> VentnSecLeYPosnReq_VentnSecLeYPosnReq/1
  AirDirecCtrlMgr_Runnable_sys/19(VentnSecRiXPosnReq_VentnSecRiXPosnReq_write) -> VentnSecRiXPosnReq_VentnSecRiXPosnReq/1
  AirDirecCtrlMgr_Runnable_sys/20(VentnSecRiYPosnReq_VentnSecRiYPosnReq_write) -> VentnSecRiYPosnReq_VentnSecRiYPosnReq/1
  AirDirecCtrlMgr_Runnable_sys/21(VGAStopDuePowerLimit_VGAStopDuePowerLimit_write) -> VGAStopDuePowerLimit_VGAStopDuePowerLimit/1
  AirDirecCtrlMgr_Runnable_sys/22(DrvrLeElecAirVentnSts_DrvrLeElecAirVentnSts_write) -> DrvrLeElecAirVentnSts_DrvrLeElecAirVentnSts/1
  AirDirecCtrlMgr_Runnable_sys/23(DrvrRiElecAirVentnSts_DrvrRiElecAirVentnSts_write) -> DrvrRiElecAirVentnSts_DrvrRiElecAirVentnSts/1
  AirDirecCtrlMgr_Runnable_sys/24(ElecAirVentnAvlSts_ElecAirVentnAvlSts_write) -> ElecAirVentnAvlSts_ElecAirVentnAvlSts/1
  AirDirecCtrlMgr_Runnable_sys/25(PassLeElecAirVentnSts_PassLeElecAirVentnSts_write) -> PassLeElecAirVentnSts_PassLeElecAirVentnSts/1
  AirDirecCtrlMgr_Runnable_sys/26(PassRiElecAirVentnSts_PassRiElecAirVentnSts_write) -> PassRiElecAirVentnSts_PassRiElecAirVentnSts/1
  AirDirecCtrlMgr_Runnable_sys/27(ReLeElecAirVentnSts_ReLeElecAirVentnSts_write) -> ReLeElecAirVentnSts_ReLeElecAirVentnSts/1
  AirDirecCtrlMgr_Runnable_sys/28(ReRiElecAirVentnSts_ReRiElecAirVentnSts_write) -> ReRiElecAirVentnSts_ReRiElecAirVentnSts/1
  AirDirecCtrlMgr_Runnable_sys/29(EleAirOutletCmpFact_EleAirOutletCmpFact_write) -> EleAirOutletCmpFact_EleAirOutletCmpFact/1
  AirDirecCtrlMgr_Runnable_sys/30(ElecVentnMotPwrCnsAct_ElecVentnMotPwrCnsAct_write) -> ElecVentnMotPwrCnsAct_ElecVentnMotPwrCnsAct/1
  AirDirecCtrlMgr_Runnable_sys/31(ElecVentnMotPwrCnsDes_ElecVentnMotPwrCnsDes_write) -> ElecVentnMotPwrCnsDes_ElecVentnMotPwrCnsDes/1
  AirDirecCtrlMgr_Runnable/1 -> AirDirecCtrlMgr_Runnable_sys/Trigger

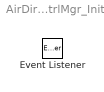
== system AirDirecCtrlMgr/AirDirecCtrlMgr_Init ==
  Event Listener : EventListener  EventName=reset
